simulink model "Sample_Model"  (R2023b)
settings: Solver=FixedStepDiscrete  StartTime=0.0  StopTime=10.0  FixedStep=1  RelTol=1e-3
settings Model: Creator=39216  LastModifiedBy=40674
settings OptimizationCC: DefaultParameterBehavior=Inlined  EnhancedBackFolding=on  PassReuseOutputArgsAs=Individual arguments  ZeroExternalMemoryAtStartup=off  ZeroInternalMemoryAtStartup=off  OptimizeBlockOrder=speed  DifferentSizesBufferReuse=on  OptimizationCustomize=off  EfficientTunableParamExpr=on
settings DebuggingCC: ParameterTunabilityLossMsg=error
settings SFSimCC: MATLABDynamicMemAlloc=off
settings RTWCC: Description=Embedded Coder  SystemTargetFile=ert.tlc  TemplateMakefile=ert_default_tmf
settings CodeAppCC: ForceParamTrailComments=on  IgnoreCustomStorageClasses=off  OperatorAnnotations=on  SimulinkDataObjDesc=on  SFDataObjDesc=on  InsertBlockDesc=on
settings ERTTargetCC: TargetLangStandard=C99 (ISO)  GenerateSampleERTMain=on  InlinedParameterPlacement=NonHierarchical  ERTCustomFileTemplate=example_file_process.tlc

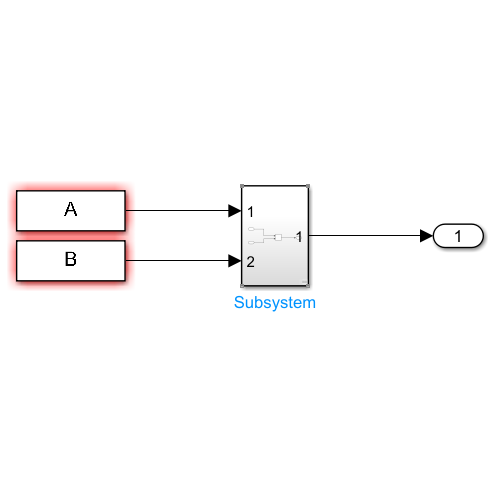
[thumbnail: Simulink's stored preview of the top-level diagram]
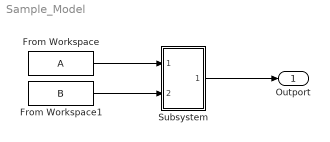
== system Sample_Model ==
  From Workspace : FromWorkspace  VariableName=A
  From Workspace1 : FromWorkspace  VariableName=B
  Subsystem : Subsystem
  Outport : Outport
  wires:
  From Workspace/1 -> Subsystem/1(Inport)
  From Workspace1/1 -> Subsystem/2(Inport1)
  Subsystem/1(Out1) -> Outport/1

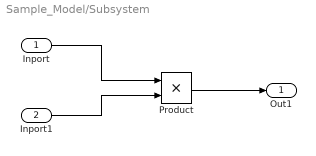
== system Sample_Model/Subsystem ==
  Inport : Inport
  Inport1 : Inport  Port=2
  Product : Product
  Out1 : Outport
  wires:
  Inport/1 -> Product/1
  Inport1/1 -> Product/2
  Product/1 -> Out1/1
